simulink model "wlan_phy_rx_pmd"  (Simulink 7.8)
settings: Solver=VariableStepDiscrete  SolverMode=SingleTasking  StartTime=0.0  StopTime=rx_sim.sim_time  RelTol=1e-3
PostLoadFcn:
  | wlan_phy_rx_init
InitFcn:
  | wlan_phy_rx_init
StartFcn:
  | wlan_phy_rx_init
values from neighbouring files (symbols this model uses):
  barker_seq20 = 0.95 * circshift(barker_seq20, [0 4]) ./ max(abs(barker_seq20))    [wlan_phy_rx_init.m]
  bit_scrambler_lfsr_bytes = sum(bit_scrambler_lfsr_bytes .* repmat(2.^[0:7], 127, 1),2)    [wlan_phy_rx_init.m]
  longCorr_coef_i = [3*real(longCorr_coef)]    [wlan_phy_rx_init.m]
  longCorr_coef_q = [3*imag(longCorr_coef)]    [wlan_phy_rx_init.m]
  MAX_CP_LEN = 32    [wlan_phy_rx_init.m]
  MAX_NCBPS = NCBPS_11n_64QAM    (= 312)    [wlan_phy_rx_init.m]
  MAX_NUM_BYTES = 2^16-1    (= 65535)    [wlan_phy_rx_init.m]
  MAX_NUM_SC = 64    [wlan_phy_rx_init.m]
  MAX_NUM_SYMS = 600    [wlan_phy_rx_init.m]
  NDBPS_11a_BPSK12 = 24    [wlan_phy_rx_init.m]
  preFFT_sampBuff_numSamps = 4*MAX_NUM_SC    (= 256)    [wlan_phy_rx_init.m]
  rx_sim = struct()    [wlan_phy_rx_init.m]
  scr_ind_rev = zeros(1,128)    [wlan_phy_rx_init.m]
undefined symbols (not defined in the file or in its folder): gcbh, mcs_rom_11ag, mcs_rom_11n, wlan_rx_deinterleave_rom_gen, xlBlockLoadCallback, xlGetNormalizedPeriod, xlipgetpartsetting
libraries: xbsIndex_r4 x4943, xbsEDKLib_r4 x1
(part 10 of 28)

== system wlan_phy_rx_pmd/DSSS Rx/Demod/Sync ==
  note: Timeout in two stages:
      |
      | A) In the first MIN_ONES_TOT bits, at least MIN_ONES bits must be 1 (the DSSS preamble is 128 1's).
      |   Timeout and reset if this fails.
      |
      | -If (A) is satisfied, search for the SFD magic number in the first BITS_TO_SFD bits. Timeout and reset
      |   if no SFD is found.
  Bit : Inport
  Bit Valid : Inport  Port=2
  Convert1 : xbsIndex_r4/Convert  gui_display_data_type=Boolean  arith_type=Boolean  n_bits=1  bin_pt=0  float_type=Single  exp_bits=8  fraction_bits=24  quantization=Truncate  overflow=Wrap  en=off  latency=0  dbl_ovrd=off  pipeline=off  block_type=convert
  Convert2 : xbsIndex_r4/Convert  gui_display_data_type=Boolean  arith_type=Boolean  n_bits=1  bin_pt=0  float_type=Single  exp_bits=8  fraction_bits=24  quantization=Truncate  overflow=Wrap  en=off  latency=0  dbl_ovrd=off  pipeline=off  block_type=convert
  Counter1 : xbsIndex_r4/Counter  cnt_type=Free Running  cnt_to=Inf  operation=Up  start_count=0  cnt_by_val=1  arith_type=Unsigned  n_bits=8  bin_pt=0  load_pin=off  rst=on  en=on  explicit_period=off  period=1  dbl_ovrd=off  use_behavioral_HDL=off  implementation=Fabric  block_type=counter
  Counter2 : xbsIndex_r4/Counter  cnt_type=Free Running  cnt_to=Inf  operation=Up  start_count=0  cnt_by_val=1  arith_type=Unsigned  n_bits=8  bin_pt=0  load_pin=off  rst=on  en=on  explicit_period=off  period=1  dbl_ovrd=off  use_behavioral_HDL=off  implementation=Fabric  block_type=counter
  DSSS Sync : Scope  NumInputPorts=7
      settings: TimeRange=56900  YMin=0~0~0~0~0~-1~-1  YMax=1~1~1~70~45~1~1  SaveName=ScopeData2
  Display : Display
  Display1 : Display
  Display2 : Display
  Down Sample1 : xbsIndex_r4/Down Sample  sample_ratio=8  sample_phase="Last Value of Frame  (most efficient)"  en=off  latency=1  dbl_ovrd=off  block_type=dsamp
  Gateway Out{,1..9} (10 alike) : xbsIndex_r4/Gateway Out  inherit_from_input=off  hdl_port=off  timing_constraint=None  locs_specified=off  LOCs={}  UseAsDAC=off  DACChannel='1'  block_type=gatewayout
  Inverter : xbsIndex_r4/Inverter  en=off  latency=0  dbl_ovrd=off  block_type=inv
      settings: xl_area=[0 0 0 0 0 0 0]  block_version=VER_STRING_GOES_HERE
  Logical : xbsIndex_r4/Logical  logical_function=AND  inputs=2  en=off  latency=0  precision=Full  arith_type=Unsigned  n_bits=8  bin_pt=2  align_bp=on  dbl_ovrd=off  block_type=logical
      settings: xl_area=[0, 0, 0, 0, 0, 0, 0]  block_version=VER_STRING_GOES_HERE
  Logical1 : xbsIndex_r4/Logical  logical_function=AND  inputs=3  en=off  latency=0  precision=Full  arith_type=Unsigned  n_bits=8  bin_pt=2  align_bp=on  dbl_ovrd=off  block_type=logical
      settings: xl_area=[0, 0, 0, 0, 0, 0, 0]  block_version=VER_STRING_GOES_HERE
  Logical2 : xbsIndex_r4/Logical  logical_function=AND  inputs=2  en=off  latency=0  precision=Full  arith_type=Unsigned  n_bits=8  bin_pt=2  align_bp=on  dbl_ovrd=off  block_type=logical
      settings: xl_area=[0, 0, 0, 0, 0, 0, 0]  block_version=VER_STRING_GOES_HERE
  Logical3 : xbsIndex_r4/Logical  logical_function=OR  inputs=2  en=off  latency=0  precision=Full  arith_type=Unsigned  n_bits=8  bin_pt=2  align_bp=on  dbl_ovrd=off  block_type=logical
      settings: xl_area=[0, 0, 0, 0, 0, 0, 0]  block_version=VER_STRING_GOES_HERE
  Logical4 : xbsIndex_r4/Logical  logical_function=AND  inputs=2  en=off  latency=0  precision=Full  arith_type=Unsigned  n_bits=8  bin_pt=2  align_bp=on  dbl_ovrd=off  block_type=logical
      settings: xl_area=[0, 0, 0, 0, 0, 0, 0]  block_version=VER_STRING_GOES_HERE
  Register8 : xbsIndex_r4/Register  init=0  rst=off  en=on  dbl_ovrd=off  block_type=register
  Relational1 : xbsIndex_r4/Relational  mode=a>b  en=off  latency=0  dbl_ovrd=off  block_type=relational
  Relational2 : xbsIndex_r4/Relational  mode=a>b  en=off  latency=0  dbl_ovrd=off  block_type=relational
  Relational3 : xbsIndex_r4/Relational  mode=a>b  en=off  latency=0  dbl_ovrd=off  block_type=relational
  S-R Latch : Subsystem
  SFD Search : Subsystem
  Payload Bit : Outport
  " Bit Valid" : Outport  Port=2
  tag scope: DSSS_RX_TIMEOUT=global
  wires:
  Bit/1 -> SFD Search/1(Bit), Convert1/1, Payload Bit/1, Register8/1
  Bit Valid/1 -> SFD Search/2(Bit Valid), Logical/2, Counter1/2, Convert2/1, Gateway Out1/1, Register8/2
  SFD Search/1(Found) -> S-R Latch/1(S)
  [DSSS_RX_RESET] -> S-R Latch/2(R)
  S-R Latch/1(Q) -> Logical/1, Inverter/1, Gateway Out4/1
  Logical/1 -> " Bit Valid"/1, Gateway Out9/1
  Counter1/1 -> Relational1/1, Relational3/1, Gateway Out5/1
  Gateway Out/1 -> Display/1
  [DSSS_RX_RESET] -> Counter1/1, Counter2/1
  Gateway Out4/1 -> DSSS Sync/3  "SFD Found"
  Gateway Out5/1 -> DSSS Sync/4  "Bit Counter"
  Gateway Out1/1 -> DSSS Sync/2  "Bit Valid"
  Down Sample1/1 -> Relational1/2
  Gateway Out2/1 -> DSSS Sync/1  "Bit"
  Logical2/1 -> Counter2/2
  [regRx_DSSS_RX_TIMEOUT_BITS_TO_SFD] -> Down Sample1/1
  Counter2/1 -> Relational2/2, Gateway Out7/1
  [regRx_DSSS_RX_TIMEOUT_MIN_ONES] -> Relational2/1
  [regRx_DSSS_RX_TIMEOUT_MIN_ONES_TOT] -> Relational3/2
  Relational3/1 -> Logical1/2
  Relational2/1 -> Logical1/3
  Logical3/1 -> [DSSS_RX_TIMEOUT], Gateway Out8/1
  Inverter/1 -> Logical1/1, Logical4/1
  Logical1/1 -> Logical3/2
  [regRx_DSSS_RX_TIMEOUT_BITS_TO_SFD] -> Gateway Out/1
  Gateway Out3/1 -> Display1/1
  [regRx_DSSS_RX_TIMEOUT_MIN_ONES_TOT] -> Gateway Out3/1
  Gateway Out6/1 -> Display2/1
  [regRx_DSSS_RX_TIMEOUT_MIN_ONES] -> Gateway Out6/1
  Gateway Out7/1 -> DSSS Sync/5  "Ones Counter"
  Gateway Out8/1 -> DSSS Sync/6  "Timed Out"
  Convert2/1 -> Logical2/1
  Convert1/1 -> Logical2/2
  Register8/1 -> Gateway Out2/1
  Logical4/1 -> Logical3/1
  Relational1/1 -> Logical4/2
  Gateway Out9/1 -> DSSS Sync/7  "Bit Valid"

== system wlan_phy_rx_pmd/DSSS Rx/Reset Gen/Posedge ==
  D : Inport
  Delay : xbsIndex_r4/Delay  rst=off  infoeditControl=Selection of Reset will increase slice count due to use of real FFs and instead of SRLs  en=off  latency=1  dbl_ovrd=off  reg_retiming=off  block_type=delay
      settings: infoedit=Hardware notes: A delay line is a chain, each link of which is an SRL16 followed by a flip-flop. If register retiming is enabled, the delay line is a chain of flip-flops.  xl_area=[1 1 0 0 0 0 0]  block_version=VER_STRING_GOES_HERE
  Inverter : xbsIndex_r4/Inverter  en=off  latency=0  dbl_ovrd=off  block_type=inv
      settings: xl_area=[0 0 0 0 0 0 0]  block_version=VER_STRING_GOES_HERE
  Logical1 : xbsIndex_r4/Logical  logical_function=AND  inputs=2  en=off  latency=0  precision=Full  arith_type=Unsigned  n_bits=8  bin_pt=2  align_bp=on  dbl_ovrd=off  block_type=logical
      settings: xl_area=[1 0 0 1 0 0 0]  block_version=VER_STRING_GOES_HERE
  Q : Outport
  wires:
  D/1 -> Logical1/2, Delay/1
  Logical1/1 -> Q/1
  Inverter/1 -> Logical1/1
  Delay/1 -> Inverter/1

== system wlan_phy_rx_pmd/DSSS Rx/Reset Gen/S-R Latch ==
  S : Inport
  R : Inport  Port=2
  Constant1 : xbsIndex_r4/Constant  const=1  gui_display_data_type=Boolean  arith_type=Boolean  n_bits=1  bin_pt=0  preci_type=Single  exp_width=8  frac_width=24  explicit_period=off  period=1  equ=P=C  opselect=C  inp2=PCIN>>17  opr=+  inp1=P  carry=CIN  dbl_ovrd=off  block_type=constant
      settings: block_version=VER_STRING_GOES_HERE
  Convert1 : xbsIndex_r4/Convert  gui_display_data_type=Boolean  arith_type=Boolean  n_bits=1  bin_pt=0  float_type=Single  exp_bits=8  fraction_bits=24  quantization=Truncate  overflow=Wrap  en=off  latency=0  dbl_ovrd=off  pipeline=off  block_type=convert
  Convert2 : xbsIndex_r4/Convert  gui_display_data_type=Boolean  arith_type=Boolean  n_bits=1  bin_pt=0  float_type=Single  exp_bits=8  fraction_bits=24  quantization=Truncate  overflow=Wrap  en=off  latency=0  dbl_ovrd=off  pipeline=off  block_type=convert
  Register2 : xbsIndex_r4/Register  init=0  rst=on  en=on  dbl_ovrd=off  block_type=register
      settings: xl_area=[1 1 0 0 0 0 0]  block_version=VER_STRING_GOES_HERE
  Q : Outport
  wires:
  Convert2/1 -> Register2/3
  Convert1/1 -> Register2/2
  Constant1/1 -> Register2/1
  R/1 -> Convert1/1
  Register2/1 -> Q/1
  S/1 -> Convert2/1

== system wlan_phy_rx_pmd/DSSS Rx/Reset Gen/S-R Latch2 ==
  S : Inport
  R : Inport  Port=2
  Constant1 : xbsIndex_r4/Constant  const=1  gui_display_data_type=Boolean  arith_type=Boolean  n_bits=1  bin_pt=0  preci_type=Single  exp_width=8  frac_width=24  explicit_period=off  period=1  equ=P=C  opselect=C  inp2=PCIN>>17  opr=+  inp1=P  carry=CIN  dbl_ovrd=off  block_type=constant
      settings: block_version=VER_STRING_GOES_HERE
  Convert1 : xbsIndex_r4/Convert  gui_display_data_type=Boolean  arith_type=Boolean  n_bits=1  bin_pt=0  float_type=Single  exp_bits=8  fraction_bits=24  quantization=Truncate  overflow=Wrap  en=off  latency=0  dbl_ovrd=off  pipeline=off  block_type=convert
  Convert2 : xbsIndex_r4/Convert  gui_display_data_type=Boolean  arith_type=Boolean  n_bits=1  bin_pt=0  float_type=Single  exp_bits=8  fraction_bits=24  quantization=Truncate  overflow=Wrap  en=off  latency=0  dbl_ovrd=off  pipeline=off  block_type=convert
  Register2 : xbsIndex_r4/Register  init=0  rst=on  en=on  dbl_ovrd=off  block_type=register
      settings: xl_area=[1 1 0 0 0 0 0]  block_version=VER_STRING_GOES_HERE
  Q : Outport
  wires:
  S/1 -> Convert2/1
  Register2/1 -> Q/1
  R/1 -> Convert1/1
  Constant1/1 -> Register2/1
  Convert1/1 -> Register2/2
  Convert2/1 -> Register2/3

== system wlan_phy_rx_pmd/DSSS Rx/Reset Gen/negedge ==
  D : Inport
  Delay : xbsIndex_r4/Delay  rst=off  infoeditControl=Selection of Reset will increase slice count due to use of real FFs and instead of SRLs  en=off  latency=1  dbl_ovrd=off  reg_retiming=off  block_type=delay
      settings: infoedit=Hardware notes: A delay line is a chain, each link of which is an SRL16 followed by a flip-flop. If register retiming is enabled, the delay line is a chain of flip-flops.  xl_area=[1 1 0 0 0 0 0]  block_version=VER_STRING_GOES_HERE
  Inverter : xbsIndex_r4/Inverter  en=off  latency=0  dbl_ovrd=off  block_type=inv
      settings: xl_area=[0 0 0 0 0 0 0]  block_version=VER_STRING_GOES_HERE
  Logical1 : xbsIndex_r4/Logical  logical_function=AND  inputs=2  en=off  latency=0  precision=Full  arith_type=Unsigned  n_bits=8  bin_pt=2  align_bp=on  dbl_ovrd=off  block_type=logical
      settings: xl_area=[1 0 0 1 0 0 0]  block_version=VER_STRING_GOES_HERE
  Q : Outport
  wires:
  Delay/1 -> Logical1/1
  Logical1/1 -> Q/1
  D/1 -> Delay/1, Inverter/1
  Inverter/1 -> Logical1/2

== system wlan_phy_rx_pmd/DSSS Rx/SIGNAL Dec/Length Check ==
  note: Require [14,1500] bytes - reject anything else
      | as invalid. Reduces wasted time attempting
      | decoding of bogus LENGTH pkts.
  Len : Inport
  Constant1 : xbsIndex_r4/Constant  const=8*14  gui_display_data_type=Fixed-point  arith_type=Unsigned  n_bits=8  bin_pt=0  preci_type=Single  exp_width=8  frac_width=24  explicit_period=off  period=1  equ=P=C  opselect=C  inp2=PCIN>>17  opr=+  inp1=P  carry=CIN  dbl_ovrd=off  block_type=constant
  Constant5 : xbsIndex_r4/Constant  const=8*1500  gui_display_data_type=Fixed-point  arith_type=Unsigned  n_bits=14  bin_pt=0  preci_type=Single  exp_width=8  frac_width=24  explicit_period=off  period=1  equ=P=C  opselect=C  inp2=PCIN>>17  opr=+  inp1=P  carry=CIN  dbl_ovrd=off  block_type=constant
  Logical9 : xbsIndex_r4/Logical  logical_function=OR  inputs=2  en=off  latency=0  precision=Full  arith_type=Unsigned  n_bits=16  bin_pt=0  align_bp=on  dbl_ovrd=off  block_type=logical
  Relational1 : xbsIndex_r4/Relational  mode=a<b  en=off  latency=0  dbl_ovrd=off  block_type=relational
  Relational5 : xbsIndex_r4/Relational  mode=a>b  en=off  latency=0  dbl_ovrd=off  block_type=relational
  Invalid : Outport
  wires:
  Constant5/1 -> Relational5/2
  Len/1 -> Relational5/1, Relational1/1
  Relational5/1 -> Logical9/1
  Constant1/1 -> Relational1/2
  Relational1/1 -> Logical9/2
  Logical9/1 -> Invalid/1

== system wlan_phy_rx_pmd/DSSS Rx/Sym Sync/Abs ==
  Valid : Inport
  I : Inport  Port=2
  Q : Inport  Port=3
  AddSub : xbsIndex_r4/AddSub  mode=Addition  use_carryin=off  use_carryout=off  en=off  latency=1  precision=Full  arith_type=Unsigned  n_bits=16  bin_pt=14  quantization=Truncate  overflow=Wrap  dbl_ovrd=off  use_behavioral_HDL=off  hw_selection=Fabric  pipelined=off  block_type=addsub
  Inverter : xbsIndex_r4/Inverter  en=off  latency=0  dbl_ovrd=off  block_type=inv
  Mult : xbsIndex_r4/Mult  precision=Full  arith_type="Signed  (2's comp)"  n_bits=16  bin_pt=14  quantization=Truncate  overflow=Wrap  en=off  latency=1  dbl_ovrd=off  use_behavioral_HDL=off  opt=Speed  use_embedded=on  optimum_pipeline=off  pipeline=on  use_rpm=on  placement_style=Triangular  block_type=mult
  Mult1 : xbsIndex_r4/Mult  precision=Full  arith_type="Signed  (2's comp)"  n_bits=16  bin_pt=14  quantization=Truncate  overflow=Wrap  en=off  latency=1  dbl_ovrd=off  use_behavioral_HDL=off  opt=Speed  use_embedded=on  optimum_pipeline=off  pipeline=on  use_rpm=on  placement_style=Triangular  block_type=mult
  Register : xbsIndex_r4/Register  init=0  rst=on  en=off  dbl_ovrd=off  block_type=register
  A : Outport
  wires:
  I/1 -> Mult/2, Mult/1
  Mult/1 -> AddSub/1
  Mult1/1 -> AddSub/2
  AddSub/1 -> Register/1
  Q/1 -> Mult1/1, Mult1/2
  Valid/1 -> Inverter/1
  Register/1 -> A/1
  Inverter/1 -> Register/2

== system wlan_phy_rx_pmd/DSSS Rx/Sym Sync/Posedge ==
  D : Inport
  Delay : xbsIndex_r4/Delay  rst=off  infoeditControl=Selection of Reset will increase slice count due to use of real FFs and instead of SRLs  en=off  latency=1  dbl_ovrd=off  reg_retiming=off  block_type=delay
      settings: infoedit=Hardware notes: A delay line is a chain, each link of which is an SRL16 followed by a flip-flop. If register retiming is enabled, the delay line is a chain of flip-flops.  xl_area=[1 1 0 0 0 0 0]  block_version=VER_STRING_GOES_HERE
  Inverter : xbsIndex_r4/Inverter  en=off  latency=0  dbl_ovrd=off  block_type=inv
      settings: xl_area=[0 0 0 0 0 0 0]  block_version=VER_STRING_GOES_HERE
  Logical1 : xbsIndex_r4/Logical  logical_function=AND  inputs=2  en=off  latency=0  precision=Full  arith_type=Unsigned  n_bits=8  bin_pt=2  align_bp=on  dbl_ovrd=off  block_type=logical
      settings: xl_area=[1 0 0 1 0 0 0]  block_version=VER_STRING_GOES_HERE
  Q : Outport
  wires:
  D/1 -> Logical1/2, Delay/1
  Logical1/1 -> Q/1
  Inverter/1 -> Logical1/1
  Delay/1 -> Inverter/1

== system wlan_phy_rx_pmd/DSSS Rx/Sym Sync/S-R Latch ==
  S : Inport
  R : Inport  Port=2
  Constant1 : xbsIndex_r4/Constant  const=1  gui_display_data_type=Fixed-point  arith_type=Boolean  n_bits=1  bin_pt=0  preci_type=Single  exp_width=8  frac_width=24  explicit_period=off  period=1  equ=P=C  opselect=C  inp2=PCIN>>17  opr=+  inp1=P  carry=CIN  dbl_ovrd=off  block_type=constant
      settings: block_version=VER_STRING_GOES_HERE
  Convert1 : xbsIndex_r4/Convert  gui_display_data_type=Boolean  arith_type=Boolean  n_bits=1  bin_pt=0  float_type=Single  exp_bits=8  fraction_bits=24  quantization=Truncate  overflow=Wrap  en=off  latency=0  dbl_ovrd=off  pipeline=off  block_type=convert
  Convert2 : xbsIndex_r4/Convert  gui_display_data_type=Boolean  arith_type=Boolean  n_bits=1  bin_pt=0  float_type=Single  exp_bits=8  fraction_bits=24  quantization=Truncate  overflow=Wrap  en=off  latency=0  dbl_ovrd=off  pipeline=off  block_type=convert
  Register2 : xbsIndex_r4/Register  init=0  rst=on  en=on  dbl_ovrd=off  block_type=register
      settings: xl_area=[1 1 0 0 0 0 0]  block_version=VER_STRING_GOES_HERE
  Q : Outport
  wires:
  Convert2/1 -> Register2/3
  Convert1/1 -> Register2/2
  Constant1/1 -> Register2/1
  R/1 -> Convert1/1
  Register2/1 -> Q/1
  S/1 -> Convert2/1

== system wlan_phy_rx_pmd/DSSS Rx/Sym Sync/Sym Match ==
  Corr : Inport
  En : Inport  Port=2
  Delay{2..5} (4 alike) : xbsIndex_r4/Delay  rst=off  infoeditControl=Selection of Reset will increase slice count due to use of real FFs and instead of SRLs  en=off  latency=10  dbl_ovrd=off  reg_retiming=off  block_type=delay
  Inverter : xbsIndex_r4/Inverter  en=off  latency=0  dbl_ovrd=off  block_type=inv
  Inverter1 : xbsIndex_r4/Inverter  en=off  latency=0  dbl_ovrd=off  block_type=inv
  Logical : xbsIndex_r4/Logical  logical_function=AND  inputs=6  en=off  latency=0  precision=Full  arith_type=Unsigned  n_bits=16  bin_pt=0  align_bp=on  dbl_ovrd=off  block_type=logical
  Sym : Outport
  wires:
  Logical/1 -> Sym/1
  Corr/1 -> Logical/1, Delay2/1
  Delay2/1 -> Inverter/1, Delay3/1
  Inverter/1 -> Logical/2
  Delay3/1 -> Logical/3, Delay4/1
  Inverter1/1 -> Logical/4
  Delay4/1 -> Inverter1/1, Delay5/1
  Delay5/1 -> Logical/5
  En/1 -> Logical/6

== system wlan_phy_rx_pmd/DSSS Rx/Sym Sync/Sym Sync Timeout ==
  Sym : Inport
  Det : Inport  Port=2
  Constant1 : xbsIndex_r4/Constant  const=20*6  gui_display_data_type=Fixed-point  arith_type=Unsigned  n_bits=8  bin_pt=0  preci_type=Single  exp_width=8  frac_width=24  explicit_period=off  period=1  equ=P=C  opselect=C  inp2=PCIN>>17  opr=+  inp1=P  carry=CIN  dbl_ovrd=off  block_type=constant
      settings: block_version=VER_STRING_GOES_HERE
  Counter1 : xbsIndex_r4/Counter  cnt_type=Free Running  cnt_to=Inf  operation=Up  start_count=0  cnt_by_val=1  arith_type=Unsigned  n_bits=8  bin_pt=0  load_pin=off  rst=on  en=on  explicit_period=off  period=1  dbl_ovrd=off  use_behavioral_HDL=off  implementation=Fabric  block_type=counter
  Down Sample1 : xbsIndex_r4/Down Sample  sample_ratio=8  sample_phase="Last Value of Frame  (most efficient)"  en=off  latency=1  dbl_ovrd=off  block_type=dsamp
  Inverter4 : xbsIndex_r4/Inverter  en=off  latency=0  dbl_ovrd=off  block_type=inv
      settings: xl_area=[0 0 0 0 0 0 0]  block_version=VER_STRING_GOES_HERE
  Logical5 : xbsIndex_r4/Logical  logical_function=AND  inputs=2  en=off  latency=0  precision=Full  arith_type=Unsigned  n_bits=16  bin_pt=0  align_bp=on  dbl_ovrd=off  block_type=logical
  Posedge : Subsystem
  Relational1 : xbsIndex_r4/Relational  mode=a>b  en=off  latency=0  dbl_ovrd=off  block_type=relational
  S-R Latch2 : Subsystem
  Timeout : Outport
  wires:
  Posedge/1(Q) -> S-R Latch2/1(S)
  S-R Latch2/1(Q) -> Down Sample1/1
  Down Sample1/1 -> Counter1/2
  Inverter4/1 -> Logical5/1
  Counter1/1 -> Relational1/1
  Constant1/1 -> Relational1/2
  Relational1/1 -> Logical5/2
  [DSSS_RX_RESET] -> Counter1/1, S-R Latch2/2(R)
  Sym/1 -> Inverter4/1
  Det/1 -> Posedge/1(D)
  Logical5/1 -> Timeout/1

== system wlan_phy_rx_pmd/Detect & Decode/De-Interleave/Mem Sel ==
  note: Simple circuit to toggle between 0 and 1 each time the En signal asserts.
  Reset : Inport
  En : Inport  Port=2
  Inverter : xbsIndex_r4/Inverter  en=off  latency=0  dbl_ovrd=off  block_type=inv
  Logical : xbsIndex_r4/Logical  logical_function=AND  inputs=2  en=off  latency=0  precision=Full  arith_type=Unsigned  n_bits=16  bin_pt=0  align_bp=on  dbl_ovrd=off  block_type=logical
  Logical1 : xbsIndex_r4/Logical  logical_function=AND  inputs=2  en=off  latency=0  precision=Full  arith_type=Unsigned  n_bits=16  bin_pt=0  align_bp=on  dbl_ovrd=off  block_type=logical
  Logical2 : xbsIndex_r4/Logical  logical_function=OR  inputs=2  en=off  latency=0  precision=Full  arith_type=Unsigned  n_bits=16  bin_pt=0  align_bp=on  dbl_ovrd=off  block_type=logical
  S-R Latch1 : Subsystem
  Rd : Outport
  Wr : Outport  Port=2
  wires:
  S-R Latch1/1(Q) -> Inverter/1, Rd/1, Logical1/2
  Logical2/1 -> S-R Latch1/2(R)
  Logical/1 -> S-R Latch1/1(S)
  Inverter/1 -> Wr/1, Logical/1
  En/1 -> Logical/2, Logical1/1
  Logical1/1 -> Logical2/1
  Reset/1 -> Logical2/2

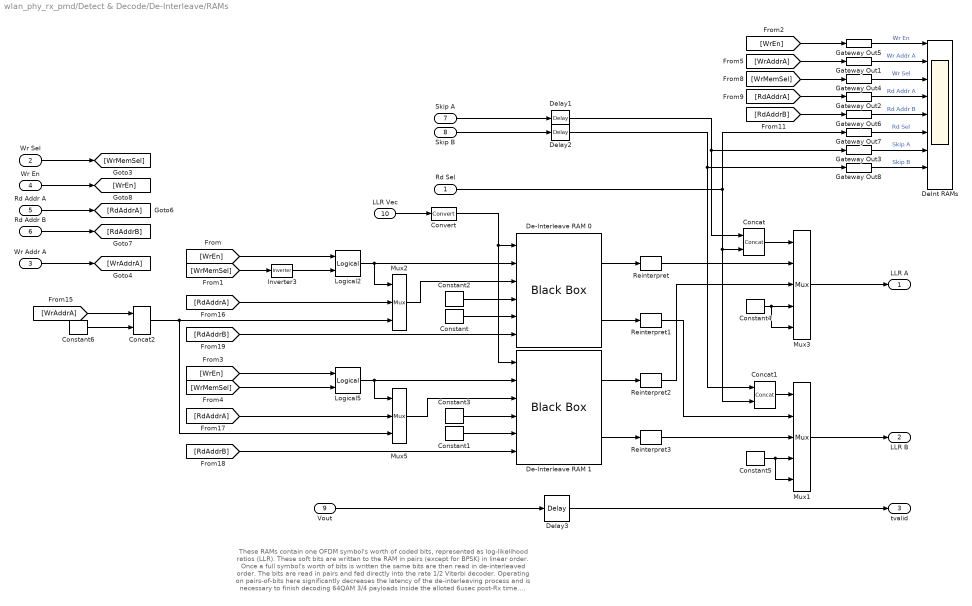
== system wlan_phy_rx_pmd/Detect & Decode/De-Interleave/RAMs ==
  note: These RAMs contain one OFDM symbol's worth of coded bits, represented as log-likelihood ratios (LLR).
      | These soft bits are written to the RAM in pairs (except for BPSK) in linear order. Once a full symbol's
      | worth of bits is written the same bits are then read in de-interleaved order. The bits are read in pairs and
      | fed directly into the rate 1/2 Viterbi decoder. Operating on pairs-of-bits here significantly decreases the latency
      | of the de-interleaving process and is necessary to finish decoding 64QAM 3/4 payloads inside the
      | alloted 6usec post-Rx time.
      | De-puncturing is realized by setting the appropriate LLR output (A or B) to 0, indicating a zero-confidence
      | value for the decoder. The Read Address Generator logic tracks which bit positions should have these
      | inserted zero-confidence values.
  Rd Sel : Inport
  Wr Sel : Inport  Port=2
  Wr Addr A : Inport  Port=3
  Wr En : Inport  Port=4
  Rd Addr A : Inport  Port=5
  Rd Addr B : Inport  Port=6
  Skip A : Inport  Port=7
  Skip B : Inport  Port=8
  Vout : Inport  Port=9
  LLR Vec : Inport  Port=10
  Concat : xbsIndex_r4/Concat  num_inputs=2  dbl_ovrd=off  block_type=concat
  Concat1 : xbsIndex_r4/Concat  num_inputs=2  dbl_ovrd=off  block_type=concat
  Concat2 : xbsIndex_r4/Concat  num_inputs=2  dbl_ovrd=off  block_type=concat
  Constant : xbsIndex_r4/Constant  const=0  gui_display_data_type=Boolean  arith_type=Boolean  n_bits=16  bin_pt=14  preci_type=Single  exp_width=8  frac_width=24  explicit_period=on  period=1  equ=P=C  opselect=C  inp2=PCIN>>17  opr=+  inp1=P  carry=CIN  dbl_ovrd=off  block_type=constant
  Constant1 : xbsIndex_r4/Constant  const=0  gui_display_data_type=Boolean  arith_type=Boolean  n_bits=16  bin_pt=14  preci_type=Single  exp_width=8  frac_width=24  explicit_period=on  period=1  equ=P=C  opselect=C  inp2=PCIN>>17  opr=+  inp1=P  carry=CIN  dbl_ovrd=off  block_type=constant
  Constant2 : xbsIndex_r4/Constant  const=0  gui_display_data_type=Fixed-point  arith_type=Unsigned  n_bits=32  bin_pt=0  preci_type=Single  exp_width=8  frac_width=24  explicit_period=on  period=1  equ=P=C  opselect=C  inp2=PCIN>>17  opr=+  inp1=P  carry=CIN  dbl_ovrd=off  block_type=constant
  Constant3 : xbsIndex_r4/Constant  const=0  gui_display_data_type=Fixed-point  arith_type=Unsigned  n_bits=32  bin_pt=0  preci_type=Single  exp_width=8  frac_width=24  explicit_period=on  period=1  equ=P=C  opselect=C  inp2=PCIN>>17  opr=+  inp1=P  carry=CIN  dbl_ovrd=off  block_type=constant
  Constant4 : xbsIndex_r4/Constant  const=0  gui_display_data_type=Fixed-point  arith_type=Signed (2's comp)  n_bits=4  bin_pt=0  preci_type=Single  exp_width=8  frac_width=24  explicit_period=on  period=1  equ=P=C  opselect=C  inp2=PCIN>>17  opr=+  inp1=P  carry=CIN  dbl_ovrd=off  block_type=constant
  Constant5 : xbsIndex_r4/Constant  const=0  gui_display_data_type=Fixed-point  arith_type=Signed (2's comp)  n_bits=4  bin_pt=0  preci_type=Single  exp_width=8  frac_width=24  explicit_period=on  period=1  equ=P=C  opselect=C  inp2=PCIN>>17  opr=+  inp1=P  carry=CIN  dbl_ovrd=off  block_type=constant
  Constant6 : xbsIndex_r4/Constant  const=0  gui_display_data_type=Fixed-point  arith_type=Unsigned  n_bits=3  bin_pt=0  preci_type=Single  exp_width=8  frac_width=24  explicit_period=off  period=1  equ=P=C  opselect=C  inp2=PCIN>>17  opr=+  inp1=P  carry=CIN  dbl_ovrd=off  block_type=constant
  Convert : xbsIndex_r4/Convert  gui_display_data_type=Fixed-point  arith_type=Unsigned  n_bits=32  bin_pt=0  float_type=Single  exp_bits=8  fraction_bits=24  quantization=Truncate  overflow=Wrap  en=off  latency=0  dbl_ovrd=off  pipeline=off  block_type=convert
  De-Interleave RAM 0 : xbsIndex_r4/Black Box  init_code=deinterleaver_ram  sim_method=ISE Simulator  verbose=off  block_type=blackbox2
  De-Interleave RAM 1 : xbsIndex_r4/Black Box  init_code=deinterleaver_ram  sim_method=ISE Simulator  verbose=off  block_type=blackbox2
  DeInt RAMs : Scope  NumInputPorts=9
      settings: TimeRange=6000  YMin=0~0~0~0~0~0~0~-10~0  YMax=1~3~1~1~300~300~1~10~1  SaveName=ScopeData49
  Delay1 : xbsIndex_r4/Delay  rst=off  infoeditControl=Selection of Reset will increase slice count due to use of real FFs and instead of SRLs  en=off  latency=1  dbl_ovrd=off  reg_retiming=off  block_type=delay
  Delay2 : xbsIndex_r4/Delay  rst=off  infoeditControl=Selection of Reset will increase slice count due to use of real FFs and instead of SRLs  en=off  latency=1  dbl_ovrd=off  reg_retiming=off  block_type=delay
  Delay3 : xbsIndex_r4/Delay  rst=off  infoeditControl=Selection of Reset will increase slice count due to use of real FFs and instead of SRLs  en=off  latency=1  dbl_ovrd=off  reg_retiming=off  block_type=delay
  Gateway Out{1..8} (8 alike) : xbsIndex_r4/Gateway Out  inherit_from_input=off  hdl_port=off  timing_constraint=None  locs_specified=off  LOCs={}  UseAsDAC=off  DACChannel='1'  block_type=gatewayout
  Inverter3 : xbsIndex_r4/Inverter  en=off  latency=0  dbl_ovrd=off  block_type=inv
  Logical2 : xbsIndex_r4/Logical  logical_function=AND  inputs=2  en=off  latency=0  precision=Full  arith_type=Unsigned  n_bits=16  bin_pt=0  align_bp=on  dbl_ovrd=off  block_type=logical
  Logical5 : xbsIndex_r4/Logical  logical_function=AND  inputs=2  en=off  latency=0  precision=Full  arith_type=Unsigned  n_bits=16  bin_pt=0  align_bp=on  dbl_ovrd=off  block_type=logical
  Mux1 : xbsIndex_r4/Mux  inputs=4  en=off  latency=0  precision=Full  arith_type=Unsigned  n_bits=16  bin_pt=14  quantization=Truncate  overflow=Wrap  dbl_ovrd=off  block_type=mux
  Mux2 : xbsIndex_r4/Mux  inputs=2  en=off  latency=0  precision=Full  arith_type=Unsigned  n_bits=16  bin_pt=14  quantization=Truncate  overflow=Wrap  dbl_ovrd=off  block_type=mux
  Mux3 : xbsIndex_r4/Mux  inputs=4  en=off  latency=0  precision=Full  arith_type=Unsigned  n_bits=16  bin_pt=14  quantization=Truncate  overflow=Wrap  dbl_ovrd=off  block_type=mux
  Mux5 : xbsIndex_r4/Mux  inputs=2  en=off  latency=0  precision=Full  arith_type=Unsigned  n_bits=16  bin_pt=14  quantization=Truncate  overflow=Wrap  dbl_ovrd=off  block_type=mux
  Reinterpret{,1..3} (4 alike) : xbsIndex_r4/Reinterpret  force_arith_type=on  arith_type="Signed  (2's comp)"  force_bin_pt=off  bin_pt=0  block_type=reinterpret
  " LLR A" : Outport
  " LLR B" : Outport  Port=2
  " tvalid" : Outport  Port=3
  wires:
  Mux3/1 -> " LLR A"/1
  Delay3/1 -> " tvalid"/1
  Vout/1 -> Delay3/1
  Logical2/1 -> Mux2/1, De-Interleave RAM 0/2
  [RdAddrA] -> Mux2/2
  Concat2/1 -> Mux2/3, Mux5/3
  Rd Addr A/1 -> [RdAddrA]
  Rd Addr B/1 -> [RdAddrB]
  Wr Addr A/1 -> [WrAddrA]
  Wr En/1 -> [WrEn]
  [WrEn] -> Logical2/1
  [WrMemSel] -> Inverter3/1
  Inverter3/1 -> Logical2/2
  Logical5/1 -> Mux5/1, De-Interleave RAM 1/2
  [WrMemSel] -> Logical5/2
  [WrEn] -> Logical5/1
  [RdAddrA] -> Mux5/2
  Wr Sel/1 -> [WrMemSel]
  Gateway Out5/1 -> DeInt RAMs/1  "Wr En"
  Gateway Out1/1 -> DeInt RAMs/2  "Wr Addr A"
  Gateway Out2/1 -> DeInt RAMs/4  "Rd Addr A"
  Gateway Out4/1 -> DeInt RAMs/3  "Wr Sel"
  Gateway Out6/1 -> DeInt RAMs/5  "Rd Addr B"
  Gateway Out7/1 -> DeInt RAMs/6  "Rd Sel"
  [WrEn] -> Gateway Out5/1
  [WrAddrA] -> Gateway Out1/1
  [WrMemSel] -> Gateway Out4/1
  [RdAddrA] -> Gateway Out2/1
  [RdAddrB] -> Gateway Out6/1
  Convert/1 -> De-Interleave RAM 0/1, De-Interleave RAM 1/1
  Constant2/1 -> De-Interleave RAM 0/4
  Constant/1 -> De-Interleave RAM 0/5
  Mux5/1 -> De-Interleave RAM 1/3
  Mux2/1 -> De-Interleave RAM 0/3
  Constant3/1 -> De-Interleave RAM 1/4
  Constant1/1 -> De-Interleave RAM 1/5
  [RdAddrB] -> De-Interleave RAM 1/6
  [RdAddrB] -> De-Interleave RAM 0/6
  De-Interleave RAM 0/1 -> Reinterpret/1
  Constant4/1 -> Mux3/4, Mux3/5
  De-Interleave RAM 1/1 -> Reinterpret2/1
  Concat/1 -> Mux3/1
  Skip A/1 -> Delay1/1
  Rd Sel/1 -> Concat/2, Concat1/2, Gateway Out7/1
  De-Interleave RAM 1/2 -> Reinterpret3/1
  De-Interleave RAM 0/2 -> Reinterpret1/1
  Concat1/1 -> Mux1/1
  Skip B/1 -> Delay2/1
  Constant5/1 -> Mux1/4, Mux1/5
  Mux1/1 -> " LLR B"/1
  Reinterpret/1 -> Mux3/2
  Reinterpret1/1 -> Mux1/2
  Reinterpret2/1 -> Mux3/3
  Reinterpret3/1 -> Mux1/3
  LLR Vec/1 -> Convert/1
  [WrAddrA] -> Concat2/1
  Constant6/1 -> Concat2/2
  Delay1/1 -> Concat/1, Gateway Out3/1
  Delay2/1 -> Concat1/1, Gateway Out8/1
  Gateway Out3/1 -> DeInt RAMs/7  "Skip A"
  Gateway Out8/1 -> DeInt RAMs/8  "Skip B"

== system wlan_phy_rx_pmd/Detect & Decode/De-Interleave/Read Addr Gen ==
  note: This is a brute force de-interleaver- the ROM stores the
      | de-interleaving pattern for each mod scheme separately.
      | The 802.11 standard interleavers have enough structure that
      | a non-ROM implementation is possible. But this design will
      | enable other interleaving patterns without changes to logic.
  Block Wr Done : Inport
  mod_sel : Inport  Port=2
  SIGNAL sym : Inport  Port=3
  rate_sel : Inport  Port=4
  Reset : Inport  Port=5
  Bus Creator : BusCreator  Inputs=2
  Bus Creator1 : BusCreator  Inputs=2
  Bus Creator2 : BusCreator  Inputs=2
  Ctrl : Subsystem
  De-Interleave DP-ROM : Subsystem
  Delay{1..4} (4 alike) : xbsIndex_r4/Delay  rst=off  infoeditControl=Selection of Reset will increase slice count due to use of real FFs and instead of SRLs  en=off  latency=1  dbl_ovrd=off  reg_retiming=off  block_type=delay
  Gateway Out{1..11} (11 alike) : xbsIndex_r4/Gateway Out  inherit_from_input=off  hdl_port=off  timing_constraint=None  locs_specified=off  LOCs={}  UseAsDAC=off  DACChannel='1'  block_type=gatewayout
  Int Rd Addr Gen : Scope  NumInputPorts=8
      settings: TimeRange=9000  YMin=-1~-1~-1~0~-0.1~-1~190~-0.1  YMax=1~1~1~1~1.1~1~217.5~1.1  SaveName=ScopeData12
  Logical5 : xbsIndex_r4/Logical  logical_function=OR  inputs=2  en=off  latency=0  precision=Full  arith_type=Unsigned  n_bits=16  bin_pt=0  align_bp=on  dbl_ovrd=off  block_type=logical
  S-R Latch1 : Subsystem
  Rd Addr A : Outport
  Rd Addr B : Outport  Port=2
  Skip A : Outport  Port=3
  Skip B : Outport  Port=4
  Vout : Outport  Port=5
  wires:
  Ctrl/5(Vout) -> Delay2/1
  Gateway Out4/1 -> Int Rd Addr Gen/3  "Count En"
  Gateway Out3/1 -> Int Rd Addr Gen/2  "Rd done"
  Gateway Out2/1 -> Int Rd Addr Gen/1  "Rate Sel"
  Gateway Out8/1 -> Int Rd Addr Gen/6  "Vout"
  Logical5/1 -> S-R Latch1/2(R), Ctrl/3(Reset)
  Block Wr Done/1 -> Delay3/1
  S-R Latch1/1(Q) -> Ctrl/4(En), Gateway Out4/1
  Reset/1 -> Logical5/2
  Delay4/1 -> Gateway Out9/1, Skip A/1
  Delay3/1 -> S-R Latch1/1(S)
  Ctrl/6(Done) -> Logical5/1, Gateway Out3/1
  Delay1/1 -> Gateway Out10/1, Skip B/1
  De-Interleave DP-ROM/1(Addr A) -> Rd Addr A/1, Gateway Out6/1
  De-Interleave DP-ROM/2(Addr B) -> Rd Addr B/1, Gateway Out11/1
  Bus Creator1/1 -> Int Rd Addr Gen/7  "Rd Addr AB"
  Gateway Out6/1 -> Bus Creator1/1
  Gateway Out11/1 -> Bus Creator1/2
  mod_sel/1 -> Ctrl/1(mod_sel)
  rate_sel/1 -> Ctrl/2(rate_sel), Gateway Out2/1
  Ctrl/1(Ind A) -> De-Interleave DP-ROM/1(Ind A), Gateway Out1/1
  Ctrl/2(Ind B) -> De-Interleave DP-ROM/2(Ind B), Gateway Out5/1
  Ctrl/3(Skip A) -> Delay4/1
  Ctrl/4(Skip B) -> Delay1/1
  Delay2/1 -> Vout/1, Gateway Out8/1
  Gateway Out9/1 -> Bus Creator/1
  Gateway Out10/1 -> Bus Creator/2
  Bus Creator/1 -> Int Rd Addr Gen/8  "Skip AB"
  Bus Creator2/1 -> Int Rd Addr Gen/4  "Rd Ind AB"
  Gateway Out1/1 -> Bus Creator2/1
  Gateway Out5/1 -> Bus Creator2/2
  SIGNAL sym/1 -> Ctrl/5(SIGNAL sym), Gateway Out7/1
  Gateway Out7/1 -> Int Rd Addr Gen/5  "SIG Sym"

== system wlan_phy_rx_pmd/Detect & Decode/De-Interleave/Write Addr Gen ==
  note: SIGNAL symbols are always 48-subcarrier BPSK 1/2. N_CBPS
      | for post-SIGNAL symbols depends on decoded RATE/MCS values.
      | "From" tag here comes from SIGNAL Decode (11a) or
      | HT-SIG Decode (11n) blocks post-decoder.
  tvalid : Inport
  SIGNAL Sym : Inport  Port=2
  Reset : Inport  Port=3
  Constant1 : xbsIndex_r4/Constant  const=51  gui_display_data_type=Fixed-point  arith_type=Unsigned  n_bits=ceil(log2(MAX_NUM_SC))  bin_pt=0  preci_type=Single  exp_width=8  frac_width=24  explicit_period=off  period=1  equ=P=C  opselect=C  inp2=PCIN>>17  opr=+  inp1=P  carry=CIN  dbl_ovrd=off  block_type=constant
  Constant10 : xbsIndex_r4/Constant  const=47  gui_display_data_type=Fixed-point  arith_type=Unsigned  n_bits=ceil(log2(MAX_NUM_SC))  bin_pt=0  preci_type=Single  exp_width=8  frac_width=24  explicit_period=off  period=1  equ=P=C  opselect=C  inp2=PCIN>>17  opr=+  inp1=P  carry=CIN  dbl_ovrd=off  block_type=constant
  Counter : xbsIndex_r4/Counter  cnt_type=Free Running  cnt_to=1  operation=Up  start_count=0  cnt_by_val=1  arith_type=Unsigned  n_bits=ceil(log2(MAX_NUM_SC))  bin_pt=0  load_pin=off  rst=on  en=on  explicit_period=off  period=1  dbl_ovrd=off  use_behavioral_HDL=off  implementation=Fabric  block_type=counter
  DeInt Wr Addr : Scope  NumInputPorts=8
      settings: TimeRange=20000  YMin=0~-0.1~0~0~0~-1~-1~-1  YMax=1~1.1~100~1~1~1~1~1  SaveName=ScopeData6
  Delay : xbsIndex_r4/Delay  rst=off  infoeditControl=Selection of Reset will increase slice count due to use of real FFs and instead of SRLs  en=off  latency=1  dbl_ovrd=off  reg_retiming=off  block_type=delay
  Gateway Out{1..5} (5 alike) : xbsIndex_r4/Gateway Out  inherit_from_input=off  hdl_port=off  timing_constraint=None  locs_specified=off  LOCs={}  UseAsDAC=off  DACChannel='1'  block_type=gatewayout
  Inverter : xbsIndex_r4/Inverter  en=off  latency=0  dbl_ovrd=off  block_type=inv
  Logical1 : xbsIndex_r4/Logical  logical_function=AND  inputs=2  en=off  latency=0  precision=Full  arith_type=Unsigned  n_bits=16  bin_pt=0  align_bp=on  dbl_ovrd=off  block_type=logical
  Logical2 : xbsIndex_r4/Logical  logical_function=OR  inputs=2  en=off  latency=0  precision=Full  arith_type=Unsigned  n_bits=16  bin_pt=0  align_bp=on  dbl_ovrd=off  block_type=logical
  Logical5 : xbsIndex_r4/Logical  logical_function=OR  inputs=2  en=off  latency=0  precision=Full  arith_type=Unsigned  n_bits=16  bin_pt=0  align_bp=on  dbl_ovrd=off  block_type=logical
  Mux4 : xbsIndex_r4/Mux  inputs=2  en=off  latency=0  precision=Full  arith_type=Unsigned  n_bits=16  bin_pt=14  quantization=Truncate  overflow=Wrap  dbl_ovrd=off  block_type=mux
  Relational1 : xbsIndex_r4/Relational  mode=a>=b  en=off  latency=0  dbl_ovrd=off  block_type=relational
  Wr Addr A : Outport
  Wr En : Outport  Port=2
  Block Wr Done : Outport  Port=3
  wires:
  Logical5/1 -> Counter/1, Gateway Out2/1
  Counter/1 -> Relational1/1, Wr Addr A/1, Gateway Out4/1
  tvalid/1 -> Counter/2, Gateway Out3/1, Wr En/1, Gateway Out1/1, Delay/1
  Relational1/1 -> Logical5/2, Logical1/1
  Reset/1 -> Logical5/1
  Gateway Out2/1 -> DeInt Wr Addr/1  "Reset"
  Gateway Out3/1 -> DeInt Wr Addr/2  "Valid In"
  Gateway Out4/1 -> DeInt Wr Addr/3  "Wr Addr A"
  Gateway Out1/1 -> DeInt Wr Addr/5  "Wr En"
  Gateway Out5/1 -> DeInt Wr Addr/6  "Block Done"
  Mux4/1 -> Relational1/2
  Logical2/1 -> Mux4/1
  Constant10/1 -> Mux4/3
  Constant1/1 -> Mux4/2
  Logical1/1 -> Block Wr Done/1, Gateway Out5/1
  Delay/1 -> Logical1/2
  SIGNAL Sym/1 -> Logical2/1
  Inverter/1 -> Logical2/2
  [RX_PHY_MODE_11N_AC] -> Inverter/1

== system wlan_phy_rx_pmd/Detect & Decode/Decode/Byte Count ==
  Byte Valid : Inport
  Decode Start : Inport  Port=2
  Last Byte Ind : Inport  Port=3
  Byte Counter : xbsIndex_r4/Counter  cnt_type=Free Running  cnt_to=1  operation=Up  start_count=0  cnt_by_val=1  arith_type=Unsigned  n_bits=ceil(log2(MAX_NUM_BYTES))  bin_pt=0  load_pin=off  rst=on  en=on  explicit_period=off  period=1  dbl_ovrd=off  use_behavioral_HDL=off  implementation=Fabric  block_type=counter
  Inverter1 : xbsIndex_r4/Inverter  en=off  latency=0  dbl_ovrd=off  block_type=inv
  Logical : xbsIndex_r4/Logical  logical_function=AND  inputs=2  en=off  latency=0  precision=Full  arith_type=Unsigned  n_bits=16  bin_pt=0  align_bp=on  dbl_ovrd=off  block_type=logical
  Logical1 : xbsIndex_r4/Logical  logical_function=OR  inputs=2  en=off  latency=0  precision=Full  arith_type=Unsigned  n_bits=16  bin_pt=0  align_bp=on  dbl_ovrd=off  block_type=logical
  Logical3 : xbsIndex_r4/Logical  logical_function=AND  inputs=2  en=off  latency=0  precision=Full  arith_type=Unsigned  n_bits=16  bin_pt=0  align_bp=on  dbl_ovrd=off  block_type=logical
  Relational2 : xbsIndex_r4/Relational  mode=a>=b  en=off  latency=0  dbl_ovrd=off  block_type=relational
  S-R Latch1 : Subsystem
  " Byte Valid" : Outport
  Byte Index : Outport  Port=2
  Last Byte : Outport  Port=3
  wires:
  Logical/1 -> Byte Counter/2, " Byte Valid"/1
  Byte Counter/1 -> Byte Index/1, Relational2/1
  Logical3/1 -> S-R Latch1/2(R), Last Byte/1
  Decode Start/1 -> S-R Latch1/1(S), Logical1/1
  S-R Latch1/1(Q) -> Logical/1, Inverter1/1
  Byte Valid/1 -> Logical/2, Logical3/1
  Relational2/1 -> Logical3/2
  Last Byte Ind/1 -> Relational2/2
  Inverter1/1 -> Logical1/2
  Logical1/1 -> Byte Counter/1

== system wlan_phy_rx_pmd/Detect & Decode/Decode/Control ==
  dec_vin : Inport
  Reset : Inport  Port=2
  Start : Inport  Port=3
  Num Bytes : Inport  Port=4
  dec_vout : Inport  Port=5
  AddSub : xbsIndex_r4/AddSub  mode=Addition  use_carryin=off  use_carryout=off  en=off  latency=0  precision=Full  arith_type=Unsigned  n_bits=16  bin_pt=14  quantization=Truncate  overflow=Wrap  dbl_ovrd=off  use_behavioral_HDL=off  hw_selection=Fabric  pipelined=off  block_type=addsub
  Coded Bit Counter : xbsIndex_r4/Counter  cnt_type=Free Running  cnt_to=1  operation=Up  start_count=0  cnt_by_val=1  arith_type=Unsigned  n_bits=3+ceil(log2(MAX_NUM_BYTES))  bin_pt=0  load_pin=off  rst=on  en=on  explicit_period=off  period=1  dbl_ovrd=off  use_behavioral_HDL=off  implementation=Fabric  block_type=counter
  Concat : xbsIndex_r4/Concat  num_inputs=2  dbl_ovrd=off  block_type=concat
  Constant1 : xbsIndex_r4/Constant  const=14  gui_display_data_type=Fixed-point  arith_type=Unsigned  n_bits=5  bin_pt=0  preci_type=Single  exp_width=8  frac_width=24  explicit_period=off  period=1  equ=P=C  opselect=C  inp2=PCIN>>17  opr=+  inp1=P  carry=CIN  dbl_ovrd=off  block_type=constant
  Constant2 : xbsIndex_r4/Constant  const=0  gui_display_data_type=Fixed-point  arith_type=Unsigned  n_bits=3  bin_pt=0  preci_type=Single  exp_width=8  frac_width=24  explicit_period=off  period=1  equ=P=C  opselect=C  inp2=PCIN>>17  opr=+  inp1=P  carry=CIN  dbl_ovrd=off  block_type=constant
  Convert : xbsIndex_r4/Convert  gui_display_data_type=Fixed-point  arith_type=Unsigned  n_bits=1  bin_pt=0  float_type=Single  exp_bits=8  fraction_bits=24  quantization=Truncate  overflow=Wrap  en=off  latency=0  dbl_ovrd=off  pipeline=off  block_type=convert
  Convert2 : xbsIndex_r4/Convert  gui_display_data_type=Fixed-point  arith_type=Unsigned  n_bits=1  bin_pt=0  float_type=Single  exp_bits=8  fraction_bits=24  quantization=Truncate  overflow=Wrap  en=off  latency=0  dbl_ovrd=off  pipeline=off  block_type=convert
  Convert7 : xbsIndex_r4/Convert  gui_display_data_type=Fixed-point  arith_type=Unsigned  n_bits=1  bin_pt=0  float_type=Single  exp_bits=8  fraction_bits=24  quantization=Truncate  overflow=Wrap  en=off  latency=0  dbl_ovrd=off  pipeline=off  block_type=convert
  Decoder Ctrl : Scope  NumInputPorts=8
      settings: TimeRange=10000  YMin=-1~-1~1998.8~0~11100~-1~-1~0  YMax=1~1~2209.2~1~11275~1~1~1  SaveName=ScopeData40
  Delay1 : xbsIndex_r4/Delay  rst=off  infoeditControl=Selection of Reset will increase slice count due to use of real FFs and instead of SRLs  en=off  latency=2  dbl_ovrd=off  reg_retiming=off  block_type=delay
  Delay2 : xbsIndex_r4/Delay  rst=off  infoeditControl=Selection of Reset will increase slice count due to use of real FFs and instead of SRLs  en=off  latency=2  dbl_ovrd=off  reg_retiming=off  block_type=delay
  Early Trace Ctrl : Subsystem
  Gateway Out{1..5,7..8,10} (8 alike) : xbsIndex_r4/Gateway Out  inherit_from_input=off  hdl_port=off  timing_constraint=None  locs_specified=off  LOCs={}  UseAsDAC=off  DACChannel='1'  block_type=gatewayout
  Inverter : xbsIndex_r4/Inverter  en=off  latency=0  dbl_ovrd=off  block_type=inv
  Logical1 : xbsIndex_r4/Logical  logical_function=AND  inputs=2  en=off  latency=0  precision=Full  arith_type=Unsigned  n_bits=16  bin_pt=0  align_bp=on  dbl_ovrd=off  block_type=logical
  Logical3 : xbsIndex_r4/Logical  logical_function=OR  inputs=2  en=off  latency=0  precision=Full  arith_type=Unsigned  n_bits=16  bin_pt=0  align_bp=on  dbl_ovrd=off  block_type=logical
  Relational2 : xbsIndex_r4/Relational  mode=a<b  en=off  latency=0  dbl_ovrd=off  block_type=relational
  negedge : Subsystem
  vin : Outport
  rst# : Outport  Port=2
  start : Outport  Port=3
  pkt_end : Outport  Port=4
  early_trace_1 : Outport  Port=5
  early_trace_2 : Outport  Port=6
  wires:
  dec_vin/1 -> Coded Bit Counter/2, Gateway Out3/1, Logical1/2
  Early Trace Ctrl/1(Early Trace 1) -> early_trace_1/1, Gateway Out5/1
  Start/1 -> Gateway Out1/1, Logical3/2, Delay1/1, Coded Bit Counter/1
  Coded Bit Counter/1 -> Gateway Out4/1, Relational2/1, Early Trace Ctrl/1(Byte Ind)
  Num Bytes/1 -> Concat/1, Gateway Out2/1
  Constant2/1 -> Concat/2
  Concat/1 -> AddSub/1
  Inverter/1 -> Convert/1
  Logical3/1 -> Inverter/1, Early Trace Ctrl/3(reset)
  Reset/1 -> Logical3/1, Gateway Out10/1
  Delay1/1 -> Convert7/1
  Convert/1 -> rst#/1
  Convert7/1 -> start/1
  Gateway Out10/1 -> Decoder Ctrl/1  "Reset"
  Gateway Out1/1 -> Decoder Ctrl/2  "Start"
  Gateway Out2/1 -> Decoder Ctrl/3  "Num Bytes"
  Gateway Out3/1 -> Decoder Ctrl/4  "Vin in"
  Gateway Out4/1 -> Decoder Ctrl/5  "Bit Counter"
  Gateway Out5/1 -> Decoder Ctrl/6  "Early Trace En"
  negedge/1(Q) -> Delay2/1
  Gateway Out7/1 -> Decoder Ctrl/7  "End"
  Logical1/1 -> Convert2/1
  Convert2/1 -> vin/1, Gateway Out8/1
  Gateway Out8/1 -> Decoder Ctrl/8  "Vin Out"
  Relational2/1 -> Logical1/1, negedge/1(D)
  Constant1/1 -> AddSub/2
  AddSub/1 -> Relational2/2
  Delay2/1 -> Gateway Out7/1, pkt_end/1
  Early Trace Ctrl/2(Early Trace 2) -> early_trace_2/1
  dec_vout/1 -> Early Trace Ctrl/2(dec_vout)

== system wlan_phy_rx_pmd/Detect & Decode/Decode/Endian Swap ==
  B : Inport
  Concat : xbsIndex_r4/Concat  num_inputs=8  dbl_ovrd=off  block_type=concat
  b0 : xbsIndex_r4/Slice  nbits=1  boolean_output=off  mode=Lower Bit Location + Width  bit1=0  base1=MSB of Input  bit0=0  base0=LSB of Input  dbl_ovrd=off  block_type=slice
  b1 : xbsIndex_r4/Slice  nbits=1  boolean_output=off  mode=Lower Bit Location + Width  bit1=0  base1=MSB of Input  bit0=1  base0=LSB of Input  dbl_ovrd=off  block_type=slice
  b2 : xbsIndex_r4/Slice  nbits=1  boolean_output=off  mode=Lower Bit Location + Width  bit1=0  base1=MSB of Input  bit0=2  base0=LSB of Input  dbl_ovrd=off  block_type=slice
  b3 : xbsIndex_r4/Slice  nbits=1  boolean_output=off  mode=Lower Bit Location + Width  bit1=0  base1=MSB of Input  bit0=3  base0=LSB of Input  dbl_ovrd=off  block_type=slice
  b4 : xbsIndex_r4/Slice  nbits=1  boolean_output=off  mode=Lower Bit Location + Width  bit1=0  base1=MSB of Input  bit0=4  base0=LSB of Input  dbl_ovrd=off  block_type=slice
  b5 : xbsIndex_r4/Slice  nbits=1  boolean_output=off  mode=Lower Bit Location + Width  bit1=0  base1=MSB of Input  bit0=5  base0=LSB of Input  dbl_ovrd=off  block_type=slice
  b6 : xbsIndex_r4/Slice  nbits=1  boolean_output=off  mode=Lower Bit Location + Width  bit1=0  base1=MSB of Input  bit0=6  base0=LSB of Input  dbl_ovrd=off  block_type=slice
  b7 : xbsIndex_r4/Slice  nbits=1  boolean_output=off  mode=Lower Bit Location + Width  bit1=0  base1=MSB of Input  bit0=7  base0=LSB of Input  dbl_ovrd=off  block_type=slice
  I : Outport
  wires:
  b7/1 -> Concat/8
  Concat/1 -> I/1
  b6/1 -> Concat/7
  b5/1 -> Concat/6
  b4/1 -> Concat/5
  b3/1 -> Concat/4
  b2/1 -> Concat/3
  b1/1 -> Concat/2
  b0/1 -> Concat/1
  B/1 -> b7/1, b6/1, b5/1, b4/1, b3/1, b2/1, b1/1, b0/1
